simulink model "Demo_TrafficFlow_cs"  (Simulink 7.6)
settings: Solver=ode5  SolverMode=SingleTasking  StartTime=0.0  StopTime=60  FixedStep=1/20  RelTol=1e-3
PreLoadFcn:
  | check_NUTS_defined
  | try
  |     evalin('base', 'NUTS_defined;');
  | catch
  |     warningMsg = sprintf('PreScan Warning: \nUsing this model requires that MATLAB is configured for PreScan. The model may not display correctly.\n\n ==> Please open MATLAB with the dedicated buttons provided with PreScan');
  |     disp(warningMsg)
  |     warndlg(warningMsg,'WindowStyle','Modal');
  | end
  | 
  | model_closed = 0;
  | assignin('base',['model_closed_' bdroot],false);
InitFcn:
  | PreScanExperimentInitFcn(bdroot)
StopFcn:
  | PreScanExperimentStopFcn(bdroot)
undefined symbols (not defined in the file or in its folder): Advised_Speed_L1, Advised_Speed_L2, Advised_Speed_L3, DiscIntNeverNeededParam, spare_10, spare_5, spare_6, spare_7, spare_8, spare_9, State_L2, Throughput_L1, Throughput_L2, Throughput_L3, Velocity_L1, Velocity_L2, Velocity_L3
libraries: simulink x58, PreScanSimulinkLibrary x40, simulink_extras x32, PreScanUsefulBlocks x22, simulink_need_slupdate x3
(part 15 of 20)

== system Demo_TrafficFlow_cs/Citroen_C3_1/AIR_3_Demux/Signal2ArrayOfSignals2 ==
  note: Set mask parameter VECTORLENGTH
      |  to MAXMESSAGES
  note: Initial state of Previous vector block
      | set to zeros(VECTORLENGTH,1)
  LoopIndex : Inport
  Element : Inport  Port=2
  Assignment : Assignment  IndexOptions=Index vector (port)  Indices=1  OutputSizes=1
  Previous vector : Memory  X0=zeros(VECTORLENGTH,1)  InheritSampleTime=on
  ArrayofElements : Outport
  wires:
  Element/1 -> Assignment/2
  LoopIndex/1 -> Assignment/3
  Previous vector/1 -> Assignment/1
  Assignment/1 -> ArrayofElements/1, Previous vector/1

== system Demo_TrafficFlow_cs/Citroen_C3_1/AIR_3_Demux/Signal2ArrayOfSignals3 ==
  note: Set mask parameter VECTORLENGTH
      |  to MAXMESSAGES
  note: Initial state of Previous vector block
      | set to zeros(VECTORLENGTH,1)
  LoopIndex : Inport
  Element : Inport  Port=2
  Assignment : Assignment  IndexOptions=Index vector (port)  Indices=1  OutputSizes=1
  Previous vector : Memory  X0=zeros(VECTORLENGTH,1)  InheritSampleTime=on
  ArrayofElements : Outport
  wires:
  Element/1 -> Assignment/2
  LoopIndex/1 -> Assignment/3
  Previous vector/1 -> Assignment/1
  Assignment/1 -> ArrayofElements/1, Previous vector/1

== system Demo_TrafficFlow_cs/Citroen_C3_1/AIR_3_Demux/Signal2ArrayOfSignals4 ==
  note: Set mask parameter VECTORLENGTH
      |  to MAXMESSAGES
  note: Initial state of Previous vector block
      | set to zeros(VECTORLENGTH,1)
  LoopIndex : Inport
  Element : Inport  Port=2
  Assignment : Assignment  IndexOptions=Index vector (port)  Indices=1  OutputSizes=1
  Previous vector : Memory  X0=zeros(VECTORLENGTH,1)  InheritSampleTime=on
  ArrayofElements : Outport
  wires:
  Element/1 -> Assignment/2
  LoopIndex/1 -> Assignment/3
  Previous vector/1 -> Assignment/1
  Assignment/1 -> ArrayofElements/1, Previous vector/1

== system Demo_TrafficFlow_cs/Citroen_C3_1/AIR_3_Demux/Signal2ArrayOfSignals5 ==
  note: Set mask parameter VECTORLENGTH
      |  to MAXMESSAGES
  note: Initial state of Previous vector block
      | set to zeros(VECTORLENGTH,1)
  LoopIndex : Inport
  Element : Inport  Port=2
  Assignment : Assignment  IndexOptions=Index vector (port)  Indices=1  OutputSizes=1
  Previous vector : Memory  X0=zeros(VECTORLENGTH,1)  InheritSampleTime=on
  ArrayofElements : Outport
  wires:
  Element/1 -> Assignment/2
  LoopIndex/1 -> Assignment/3
  Previous vector/1 -> Assignment/1
  Assignment/1 -> ArrayofElements/1, Previous vector/1

== system Demo_TrafficFlow_cs/Citroen_C3_1/AIR_3_Demux/Signal2ArrayOfSignals6 ==
  note: Set mask parameter VECTORLENGTH
      |  to MAXMESSAGES
  note: Initial state of Previous vector block
      | set to zeros(VECTORLENGTH,1)
  LoopIndex : Inport
  Element : Inport  Port=2
  Assignment : Assignment  IndexOptions=Index vector (port)  Indices=1  OutputSizes=1
  Previous vector : Memory  X0=zeros(VECTORLENGTH,1)  InheritSampleTime=on
  ArrayofElements : Outport
  wires:
  Element/1 -> Assignment/2
  LoopIndex/1 -> Assignment/3
  Previous vector/1 -> Assignment/1
  Assignment/1 -> ArrayofElements/1, Previous vector/1

== system Demo_TrafficFlow_cs/Citroen_C3_1/RFOBU_3_Rx_Demux/Reorder ==
  In : Inport
  Selector1 : Selector  InputPortWidth=InputPortWidth  IndexOptions=Index vector (dialog)  Indices=Elements  OutputSizes=1
  Out : Outport
  wires:
  In/1 -> Selector1/1
  Selector1/1 -> Out/1

== system Demo_TrafficFlow_cs/Citroen_C3_1/SELF_Demux/compas converter ==
  rotz [rad] : Inport
  cart2compas : Subsystem
  rad2deg : Gain  Gain=180/pi
  heading [deg] : Outport
  wires:
  cart2compas/1(heading [deg]) -> heading [deg]/1
  rotz [rad]/1 -> rad2deg/1
  rad2deg/1 -> cart2compas/1(Angle [deg])

== system Demo_TrafficFlow_cs/Citroen_C3_1/Simple_Longitudinal_ACC/HOLD Advised Speed ==
  v : Inport
  Memory : Memory  X0=50
  Switch : Switch  Criteria=u2 > Threshold
  v_hold : Outport
  wires:
  v/1 -> Switch/1, Switch/2
  Memory/1 -> Switch/3
  Switch/1 -> v_hold/1, Memory/1

== system Demo_TrafficFlow_cs/Citroen_C3_1/Simple_Longitudinal_ACC/LONGITUDINAL ACC & Dynamics ==
  note: DYNAMICS
  note: CONTROL
  note: CASE: DETECTION
      | vehicle in front detected
      | * postion control
      | * velocity control
  note: CASE: NO DETECTION
      | no vehicle in front detected
      | * velocity control
  Detected Range [m] : Inport
  Detected Velocity Vehicle [m/s] : Inport  Port=2
  Own Velocity Intention [km/h] : Inport  Port=3
  Safety Distance [m] : Inport  Port=4
  Gain1 : Gain  Gain=0.35
  Gain2 : Gain  Gain=1/3.6
  Rate Limiter2 : RateLimiter  RisingSlewLimit=3  FallingSlewLimit=-3  SampleTimeMode=inherited
  Sum : Sum  Inputs=-+|
  Sum2 : Sum  Inputs=++|
  Switch : Switch  Threshold=0.0001
  only forward driving : Saturate  UpperLimit=inf  LowerLimit=0
  Velocity [m/s] : Outport
  wires:
  Rate Limiter2/1 -> only forward driving/1
  only forward driving/1 -> Velocity [m/s]/1
  Sum/1 -> Gain1/1  "Control Error"
  Switch/1 -> Rate Limiter2/1
  Detected Range [m]/1 -> Switch/2, Sum/2
  Own Velocity Intention [km/h]/1 -> Gain2/1
  Gain2/1 -> Switch/3
  Sum2/1 -> Switch/1
  Detected Velocity Vehicle [m/s]/1 -> Sum2/2
  Safety Distance [m]/1 -> Sum/1
  Gain1/1 -> Sum2/1

== system Demo_TrafficFlow_cs/Citroen_C3_1/Trajectories/END OF TRACK REACHED & GO TO BUFFER ==
  Elapsed_Track [m] : Inport
  Length_of_Track [m] : Inport  Port=2
  Product1 : Product  RndMeth=Zero
  Relational Operator : RelationalOperator  OutDataTypeStr=boolean
  offset for displacement to  buffer of traffic LANE 3 : Constant  Tag=TrajSubSelector  Value=[0 27 0 0 0 0 0 0 0]
  Buffer_offset [m] : Outport
  End_of_Track_Reached [-] : Outport  Port=2
  wires:
  offset for displacement to  buffer of traffic LANE 3/1 -> Product1/1
  Relational Operator/1 -> Product1/2, End_of_Track_Reached [-]/1
  Elapsed_Track [m]/1 -> Relational Operator/1
  Product1/1 -> Buffer_offset [m]/1
  Length_of_Track [m]/1 -> Relational Operator/2

== system Demo_TrafficFlow_cs/Citroen_C3_1/Trajectories/ElapsedTrack1 ==
  Offset : Inport
  s : Inport  Port=2
  Mux : Mux  Inputs=3
  Mux1 : Mux  Inputs=3
  MuxState : Subsystem
  OffsetCor : Subsystem
  pitchAngle : Lookup  InputValues=s  Table=pitch  LookUpMeth=Interpolation-Use End Values
  rollAngle : Lookup  InputValues=s  Table=roll  LookUpMeth=Interpolation-Use End Values
  xpos : Lookup  InputValues=s  Table=x  LookUpMeth=Interpolation-Use End Values
  yawAngle : Lookup  InputValues=s  Table=yaw  LookUpMeth=Interpolation-Use End Values
  ypos : Lookup  InputValues=s  Table=y  LookUpMeth=Interpolation-Use End Values
  zpos : Lookup  InputValues=s  Table=z  LookUpMeth=Interpolation-Use End Values
  state : Outport
  wires:
  zpos/1 -> OffsetCor/3(z pos)
  rollAngle/1 -> OffsetCor/4(R angle)
  pitchAngle/1 -> OffsetCor/5(P angle)
  yawAngle/1 -> OffsetCor/6(Y angle)
  OffsetCor/6(Y angle_cor) -> Mux1/3
  OffsetCor/5(P angle_cor) -> Mux1/2
  OffsetCor/4(R angle_cor) -> Mux1/1
  OffsetCor/3(z pos_cor) -> Mux/3
  MuxState/1(State) -> state/1
  Mux/1 -> MuxState/1(xyzPos_[m])
  s/1 -> pitchAngle/1, yawAngle/1, rollAngle/1, zpos/1, ypos/1, xpos/1
  Offset/1 -> OffsetCor/7(Offset)
  xpos/1 -> OffsetCor/1(x pos)
  ypos/1 -> OffsetCor/2(y pos)
  OffsetCor/1(x pos_cor) -> Mux/1
  OffsetCor/2(y pos_cor) -> Mux/2
  Mux1/1 -> MuxState/2(RPYAngle_[rad])

== system Demo_TrafficFlow_cs/Citroen_C3_1/Trajectories/LANE CHANGE LEFT (cummulative y position;  multiple shift to left are possible) ==
  reset cum value : Inport
  velocity [m/s] : Inport  Port=2
  on/off [-] : Inport  Port=3
  Cumulative Lane Change : Subsystem
  Single LaneChange_Left : Subsystem
  Trigger : Subsystem  TreatAsAtomicUnit=on
  cum LC : Outport
  wires:
  on/off [-]/1 -> Single LaneChange_Left/1(on/off [-]), Trigger/Trigger  "lane_change_signal"
  Single LaneChange_Left/1(end LC reached) -> Cumulative Lane Change/2(end LC reached)  "end_reached"
  Trigger/1(Out1) -> Single LaneChange_Left/Enable
  velocity [m/s]/1 -> Single LaneChange_Left/2(velocity [m/s])
  reset cum value/1 -> Cumulative Lane Change/1(reset cum value)  "reset_signal"
  Single LaneChange_Left/2(single LC value) -> Cumulative Lane Change/3(single LC value)  "single LC value"
  Cumulative Lane Change/1(cum LC value) -> cum LC/1

== system Demo_TrafficFlow_cs/Citroen_C3_1/Trajectories/PathFollowerSva_3 ==
  From Workspace : FromWorkspace  VariableName=trajectory  SampleTime=TimeStep  OutputAfterFinalValue=Holding final value
  From Workspace1 : FromWorkspace  VariableName=trajectory  SampleTime=TimeStep  OutputAfterFinalValue=Setting to zero
  Mux : Mux  Inputs=2
  Selector1 : Selector  InputPortWidth=24  IndexOptions=Index vector (dialog)  Indices=[7:18]  OutputSizes=1
  Selector9 : Selector  InputPortWidth=24  IndexOptions=Index vector (dialog)  Indices=[1:6]  OutputSizes=1
  trajectory : Outport
  wires:
  Selector1/1 -> Mux/2
  Selector9/1 -> Mux/1
  Mux/1 -> trajectory/1
  From Workspace1/1 -> Selector1/1
  From Workspace/1 -> Selector9/1

== system Demo_TrafficFlow_cs/Citroen_C3_1/Trajectories/RESTART TRAJECT ==
  End_of_Track_Reached [-] : Inport
  ID_start_again [-] : Inport  Port=2
  objectID [-] : Inport  Port=3
  Logical Operator : Logic  AllPortsSameDT=off  OutDataTypeStr=boolean
  Memory : Memory
  Relational Operator1 : RelationalOperator  Operator===  OutDataTypeStr=boolean
  reset [-] : Outport
  Start_Traject [-] : Outport  Port=2
  wires:
  objectID [-]/1 -> Relational Operator1/2
  Logical Operator/1 -> Memory/1
  End_of_Track_Reached [-]/1 -> Logical Operator/1
  Memory/1 -> Start_Traject [-]/1
  Relational Operator1/1 -> Logical Operator/2, reset [-]/1
  ID_start_again [-]/1 -> Relational Operator1/1

== system Demo_TrafficFlow_cs/Citroen_C3_1/Trajectories/Random  LC generator ==
  Random Number : RandomNumber  Mean=0.5  Variance=0.1  SampleTime=0
  Rounding Function : Rounding  Operator=round
  0/1 : Outport
  wires:
  Random Number/1 -> Rounding Function/1
  Rounding Function/1 -> 0/1/1

== system Demo_TrafficFlow_cs/Citroen_C3_1/Trajectories/Traj2State1 ==
  Traj Org : Inport
  Offset : Inport  Port=2
  Add2 : Sum
  ApplyRotation : PreScanSimulinkLibrary/ApplyRotation  SystemSampleTime=-1
  ApplyRotation1 : PreScanSimulinkLibrary/ApplyRotation  SystemSampleTime=-1
  ApplyRotation2 : PreScanSimulinkLibrary/ApplyRotation  SystemSampleTime=-1
  Constant : Constant  Value=zeros(12,1)
  Mux : Mux  Inputs=6
  Mux1 : Mux  Inputs=2
  Mux{2,4..9} (7 alike) : Mux  Inputs=3
  Mux3 : Mux  Inputs=2
  Selector : Selector  InputPortWidth=6  IndexOptions=Index vector (dialog)  Indices=[4 5 6]  OutputSizes=1
  Selector1 : Selector  InputPortWidth=21  IndexOptions=Index vector (dialog)  Indices=[5]  OutputSizes=1
  Selector10 : Selector  InputPortWidth=21  IndexOptions=Index vector (dialog)  Indices=[2]  OutputSizes=1
  Selector11 : Selector  InputPortWidth=21  IndexOptions=Index vector (dialog)  Indices=[3]  OutputSizes=1
  Selector12 : Selector  InputPortWidth=21  IndexOptions=Index vector (dialog)  Indices=[1]  OutputSizes=1
  Selector13 : Selector  InputPortWidth=21  IndexOptions=Index vector (dialog)  Indices=[8]  OutputSizes=1
  Selector14 : Selector  InputPortWidth=21  IndexOptions=Index vector (dialog)  Indices=[9]  OutputSizes=1
  Selector15 : Selector  InputPortWidth=21  IndexOptions=Index vector (dialog)  Indices=[7]  OutputSizes=1
  Selector16 : Selector  InputPortWidth=21  IndexOptions=Index vector (dialog)  Indices=[14]  OutputSizes=1
  Selector17 : Selector  InputPortWidth=21  IndexOptions=Index vector (dialog)  Indices=[15]  OutputSizes=1
  Selector18 : Selector  InputPortWidth=21  IndexOptions=Index vector (dialog)  Indices=[13]  OutputSizes=1
  Selector19 : Selector  InputPortWidth=21  IndexOptions=Index vector (dialog)  Indices=[20]  OutputSizes=1
  Selector2 : Selector  InputPortWidth=21  IndexOptions=Index vector (dialog)  Indices=[6]  OutputSizes=1
  Selector20 : Selector  InputPortWidth=21  IndexOptions=Index vector (dialog)  Indices=[21]  OutputSizes=1
  Selector21 : Selector  InputPortWidth=21  IndexOptions=Index vector (dialog)  Indices=[19]  OutputSizes=1
  Selector3 : Selector  InputPortWidth=21  IndexOptions=Index vector (dialog)  Indices=[12]  OutputSizes=1
  Selector4 : Selector  InputPortWidth=21  IndexOptions=Index vector (dialog)  Indices=[17]  OutputSizes=1
  Selector5 : Selector  InputPortWidth=21  IndexOptions=Index vector (dialog)  Indices=[18]  OutputSizes=1
  Selector6 : Selector  InputPortWidth=21  IndexOptions=Index vector (dialog)  Indices=[16]  OutputSizes=1
  Selector7 : Selector  InputPortWidth=21  IndexOptions=Index vector (dialog)  Indices=[11]  OutputSizes=1
  Selector8 : Selector  InputPortWidth=21  IndexOptions=Index vector (dialog)  Indices=[10]  OutputSizes=1
  Selector9 : Selector  InputPortWidth=21  IndexOptions=Index vector (dialog)  Indices=[4]  OutputSizes=1
  Traj2State2 : Subsystem
  state : Outport
  wires:
  Mux9/1 -> ApplyRotation2/2, ApplyRotation1/2, ApplyRotation/2
  Selector20/1 -> Mux9/3
  Selector19/1 -> Mux9/2
  Selector21/1 -> Mux9/1
  Selector5/1 -> Mux8/3
  Selector4/1 -> Mux8/2
  Selector6/1 -> Mux8/1
  Mux8/1 -> Mux/6
  Mux6/1 -> ApplyRotation2/1
  Mux7/1 -> Mux/4
  ApplyRotation2/1 -> Mux/5
  Selector3/1 -> Mux7/3
  Selector7/1 -> Mux7/2
  Selector8/1 -> Mux7/1
  Mux4/1 -> Mux/2
  Mux5/1 -> ApplyRotation1/1
  ApplyRotation1/1 -> Mux/3
  Mux2/1 -> ApplyRotation/1
  ApplyRotation/1 -> Mux/1
  Selector2/1 -> Mux4/3
  Selector1/1 -> Mux4/2
  Selector9/1 -> Mux4/1
  Selector17/1 -> Mux6/3
  Selector16/1 -> Mux6/2
  Selector18/1 -> Mux6/1
  Selector14/1 -> Mux5/3
  Selector13/1 -> Mux5/2
  Selector15/1 -> Mux5/1
  Selector11/1 -> Mux2/3
  Selector10/1 -> Mux2/2
  Selector12/1 -> Mux2/1
  Mux3/1 -> Selector8/1, Selector7/1, Selector3/1, Selector18/1, Selector16/1, Selector17/1, Selector6/1, Selector20/1, Selector19/1, Selector21/1, Selector5/1, Selector4/1, Selector14/1, Selector13/1, Selector15/1, Selector2/1, Selector1/1, Selector9/1, Selector12/1, Selector10/1, Selector11/1
  Mux1/1 -> Add2/2
  Constant/1 -> Mux1/2
  Add2/1 -> Traj2State2/1(traj)
  Selector/1 -> Mux3/2
  Traj Org/1 -> Mux3/1
  Offset/1 -> Mux1/1, Selector/1
  Mux/1 -> Add2/1
  Traj2State2/1(state) -> state/1

== system Demo_TrafficFlow_cs/Control_Unit/Antenna_Rx_7_Demux/Reorder ==
  In : Inport
  Selector1 : Selector  InputPortWidth=InputPortWidth  IndexOptions=Index vector (dialog)  Indices=Elements  OutputSizes=1
  Out : Outport
  wires:
  Selector1/1 -> Out/1
  In/1 -> Selector1/1

== system Demo_TrafficFlow_cs/Control_Unit/Antenna_Rx_7_Demux/compas converter ==
  rotz [rad] : Inport
  cart2compas : Subsystem
  rad2deg : Gain  Gain=180/pi
  heading [deg] : Outport
  wires:
  rad2deg/1 -> cart2compas/1(Angle [deg])
  rotz [rad]/1 -> rad2deg/1
  cart2compas/1(heading [deg]) -> heading [deg]/1

== system Demo_TrafficFlow_cs/Control_Unit/Antenna_Rx_8_Demux/Reorder ==
  In : Inport
  Selector1 : Selector  InputPortWidth=InputPortWidth  IndexOptions=Index vector (dialog)  Indices=Elements  OutputSizes=1
  Out : Outport
  wires:
  Selector1/1 -> Out/1
  In/1 -> Selector1/1

== system Demo_TrafficFlow_cs/Control_Unit/Antenna_Rx_8_Demux/compas converter ==
  rotz [rad] : Inport
  cart2compas : Subsystem
  rad2deg : Gain  Gain=180/pi
  heading [deg] : Outport
  wires:
  rad2deg/1 -> cart2compas/1(Angle [deg])
  rotz [rad]/1 -> rad2deg/1
  cart2compas/1(heading [deg]) -> heading [deg]/1

== system Demo_TrafficFlow_cs/Control_Unit/Antenna_Rx_9_Demux/Reorder ==
  In : Inport
  Selector1 : Selector  InputPortWidth=InputPortWidth  IndexOptions=Index vector (dialog)  Indices=Elements  OutputSizes=1
  Out : Outport
  wires:
  Selector1/1 -> Out/1
  In/1 -> Selector1/1

== system Demo_TrafficFlow_cs/Control_Unit/Antenna_Rx_9_Demux/compas converter ==
  rotz [rad] : Inport
  cart2compas : Subsystem
  rad2deg : Gain  Gain=180/pi
  heading [deg] : Outport
  wires:
  rad2deg/1 -> cart2compas/1(Angle [deg])
  rotz [rad]/1 -> rad2deg/1
  cart2compas/1(heading [deg]) -> heading [deg]/1

== system Demo_TrafficFlow_cs/Control_Unit/Control Lane2/Lane2 Closed ==
  IfAction : ActionPort  PropagateVarSize=During execution  ActionType=else
  State: Lane2 Closed : Constant  Value=0
  Out : Outport  OutDataTypeStr=double  InitialOutput=[0]
  wires:
  State: Lane2 Closed/1 -> Out/1

== system Demo_TrafficFlow_cs/Control_Unit/Control Lane2/Lane2 open for Lane1 ==
  note: To make sure that the lane is empty,
      | a delay is applied based on advised
      | velocity
  Close time : Inport
  IfAction : ActionPort  InitializeStates=reset  PropagateVarSize=During execution  ActionType=then
  "Close Lane2 for X seconds " : RelationalOperator  OutDataTypeStr=boolean
  Counter : Subsystem
  Product : Product  RndMeth=Zero
  State: Lane2 open for Lane1 : Constant
  Out : Outport  InitialOutput=[1]
  wires:
  Product/1 -> Out/1
  State: Lane2 open for Lane1/1 -> Product/1
  Counter/1(Out1) -> "Close Lane2 for X seconds "/1
  "Close Lane2 for X seconds "/1 -> Product/2
  Close time/1 -> "Close Lane2 for X seconds "/2

== system Demo_TrafficFlow_cs/Control_Unit/Control Lane2/Lane2 open for Lane3 ==
  note: To make sure that the lane is empty,
      | a delay is applied based on advised
      | velocity
  Close time : Inport
  IfAction : ActionPort  InitializeStates=reset  PropagateVarSize=During execution  ActionType=elseif
  "Close Lane2 for X seconds " : RelationalOperator  OutDataTypeStr=boolean
  Counter : Subsystem
  Product : Product  RndMeth=Zero
  Scope : Scope  NumInputPorts=3
      settings: YMin=-5~-5~-5  YMax=5~5~5
  State: Lane2 open for Lane3 : Constant  Value=3
  Out : Outport  InitialOutput=[3]
  wires:
  "Close Lane2 for X seconds "/1 -> Product/2, Scope/2
  Product/1 -> Out/1
  Counter/1(Out1) -> "Close Lane2 for X seconds "/1, Scope/3
  State: Lane2 open for Lane3/1 -> Product/1
  Close time/1 -> "Close Lane2 for X seconds "/2, Scope/1

== system Demo_TrafficFlow_cs/HighwayOverhang_1/Antenna_Rx_2_Demux/Reorder ==
  In : Inport
  Selector1 : Selector  InputPortWidth=InputPortWidth  IndexOptions=Index vector (dialog)  Indices=Elements  OutputSizes=1
  Out : Outport
  wires:
  Selector1/1 -> Out/1
  In/1 -> Selector1/1

== system Demo_TrafficFlow_cs/HighwayOverhang_1/Antenna_Rx_2_Demux/compas converter ==
  rotz [rad] : Inport
  cart2compas : Subsystem
  rad2deg : Gain  Gain=180/pi
  heading [deg] : Outport
  wires:
  rad2deg/1 -> cart2compas/1(Angle [deg])
  rotz [rad]/1 -> rad2deg/1
  cart2compas/1(heading [deg]) -> heading [deg]/1

== system Demo_TrafficFlow_cs/HighwayOverhang_1/RFBeacon_1_Rx_Demux/Reorder ==
  In : Inport
  Selector1 : Selector  InputPortWidth=InputPortWidth  IndexOptions=Index vector (dialog)  Indices=Elements  OutputSizes=1
  Out : Outport
  wires:
  Selector1/1 -> Out/1
  In/1 -> Selector1/1

== system Demo_TrafficFlow_cs/HighwayOverhang_1/RFBeacon_1_Rx_Demux/compas converter ==
  rotz [rad] : Inport
  cart2compas : Subsystem
  rad2deg : Gain  Gain=180/pi
  heading [deg] : Outport
  wires:
  rad2deg/1 -> cart2compas/1(Angle [deg])
  rotz [rad]/1 -> rad2deg/1
  cart2compas/1(heading [deg]) -> heading [deg]/1

== system Demo_TrafficFlow_cs/HighwayOverhang_1/Throughput detection  Sensor/TrafficDensityCounter [cars/hour] ==
  Detection : Inport
  Velocity [m/s] : Inport  Port=2
  Gain1 : Gain  Gain=60/MT
  Gain2 : Gain  Gain=1/MT
  Gain3 : Gain  Gain=60*60/MT
  Increment Counter : Sum  Inputs=+-
  Memory : Memory
  Product1 : Product  RndMeth=Zero
  Product2 : Product  RndMeth=Zero
  Rate Transition : RateTransition
  Rate Transition1 : RateTransition  OutPortSampleTime=1/20
  Ref_Range1 : Constant  Value=0
  Relational Operator : RelationalOperator  Operator=>  OutDataTypeStr=boolean
  Relational Operator1 : RelationalOperator  Operator=~=  OutDataTypeStr=boolean
  Step1 : Step  Time=MT*0  SampleTime=0
  Subsystem : Subsystem
  Subsystem1 : Subsystem
  Transport Delay1 : TransportDelay  DelayTime=MT
  Weighted Moving Average : simulink_need_slupdate/Weighted Moving Average  mgainval=ones(1,MT*20)/(MT*20)  vinit=0.0  samptime=1/20  OutDataType=sfix(16)  OutScaling=2^-10  DoSatur=off  GainDataType=sfix( 16 )  MatRadixGroup=Best Precision: Matrix-wise  GainScaling=2^-10
  Out_[1/MT]_unweighted : Outport
  Out_[1/MT] : Outport  Port=2
  Out_[1/s] : Outport  Port=3
  Out_[1/min] : Outport  Port=4
  Out_[1/hour] : Outport  Port=5
  Velocity [km/h] : Outport  Port=6
  wires:
  Ref_Range1/1 -> Relational Operator/2
  Detection/1 -> Relational Operator/1, Relational Operator1/2, Memory/1
  Subsystem/1(Out1) -> Transport Delay1/1, Increment Counter/1
  Transport Delay1/1 -> Increment Counter/2
  Increment Counter/1 -> Product1/2
  Step1/1 -> Product1/1
  Product1/1 -> Rate Transition1/1, Out_[1/MT]_unweighted/1
  Relational Operator1/1 -> Product2/1
  Relational Operator/1 -> Product2/2
  Memory/1 -> Relational Operator1/1
  Product2/1 -> Subsystem/Enable, Subsystem1/Enable
  Weighted Moving Average/1 -> Rate Transition/1
  Rate Transition/1 -> Out_[1/MT]/1, Gain2/1, Gain1/1, Gain3/1
  Rate Transition1/1 -> Weighted Moving Average/1
  Gain2/1 -> Out_[1/s]/1
  Gain1/1 -> Out_[1/min]/1
  Gain3/1 -> Out_[1/hour]/1
  Velocity [m/s]/1 -> Subsystem1/1(Velocity [m/s])
  Subsystem1/1(Velocity [km/h]) -> Velocity [km/h]/1

== system Demo_TrafficFlow_cs/HighwayOverhang_2/Antenna_Rx_3_Demux/Reorder ==
  In : Inport
  Selector1 : Selector  InputPortWidth=InputPortWidth  IndexOptions=Index vector (dialog)  Indices=Elements  OutputSizes=1
  Out : Outport
  wires:
  Selector1/1 -> Out/1
  In/1 -> Selector1/1

== system Demo_TrafficFlow_cs/HighwayOverhang_2/Antenna_Rx_3_Demux/compas converter ==
  rotz [rad] : Inport
  cart2compas : Subsystem
  rad2deg : Gain  Gain=180/pi
  heading [deg] : Outport
  wires:
  rad2deg/1 -> cart2compas/1(Angle [deg])
  rotz [rad]/1 -> rad2deg/1
  cart2compas/1(heading [deg]) -> heading [deg]/1

== system Demo_TrafficFlow_cs/HighwayOverhang_2/Control Lane2/Lane2 Closed ==
  IfAction : ActionPort  PropagateVarSize=During execution  ActionType=else
  Closed for Lane 3 : Constant  Value=0
  Closed for Lane1 : Constant  Value=0
  Mux : Mux  Inputs=2
  Out : Outport  InitialOutput=[0 0]
  wires:
  Mux/1 -> Out/1
  Closed for Lane1/1 -> Mux/1
  Closed for Lane 3/1 -> Mux/2

== system Demo_TrafficFlow_cs/HighwayOverhang_2/Control Lane2/Lane2 open for Lane1 ==
  IfAction : ActionPort  InitializeStates=reset  PropagateVarSize=During execution  ActionType=then
  Closed for Lane 3 : Constant  Value=0
  Mux : Mux  Inputs=2
  Open for Lane1 : Constant
  Out : Outport  InitialOutput=[1 0 ]
  wires:
  Mux/1 -> Out/1
  Open for Lane1/1 -> Mux/1
  Closed for Lane 3/1 -> Mux/2

== system Demo_TrafficFlow_cs/HighwayOverhang_2/Control Lane2/Lane2 open for Lane3 ==
  IfAction : ActionPort  InitializeStates=reset  PropagateVarSize=During execution  ActionType=elseif
  Closed for Lane1 : Constant  Value=0
  Mux : Mux  Inputs=2
  Open for Lane 3 : Constant
  Out : Outport  InitialOutput=[0 1]
  wires:
  Mux/1 -> Out/1
  Closed for Lane1/1 -> Mux/1
  Open for Lane 3/1 -> Mux/2

== system Demo_TrafficFlow_cs/HighwayOverhang_2/RFBeacon_2_Rx_Demux/Reorder ==
  In : Inport
  Selector1 : Selector  InputPortWidth=InputPortWidth  IndexOptions=Index vector (dialog)  Indices=Elements  OutputSizes=1
  Out : Outport
  wires:
  Selector1/1 -> Out/1
  In/1 -> Selector1/1

== system Demo_TrafficFlow_cs/HighwayOverhang_2/RFBeacon_2_Rx_Demux/compas converter ==
  rotz [rad] : Inport
  cart2compas : Subsystem
  rad2deg : Gain  Gain=180/pi
  heading [deg] : Outport
  wires:
  rad2deg/1 -> cart2compas/1(Angle [deg])
  rotz [rad]/1 -> rad2deg/1
  cart2compas/1(heading [deg]) -> heading [deg]/1

== system Demo_TrafficFlow_cs/HighwayOverhang_2/Throughput detection  Sensor/TrafficDensityCounter [cars/hour] ==
  Detection : Inport
  Velocity [m/s] : Inport  Port=2
  Gain1 : Gain  Gain=60/MT
  Gain2 : Gain  Gain=1/MT
  Gain3 : Gain  Gain=60*60/MT
  Increment Counter : Sum  Inputs=+-
  Memory : Memory
  Product1 : Product  RndMeth=Zero
  Product2 : Product  RndMeth=Zero
  Rate Transition : RateTransition
  Rate Transition1 : RateTransition  OutPortSampleTime=1/20
  Ref_Range1 : Constant  Value=0
  Relational Operator : RelationalOperator  Operator=>  OutDataTypeStr=boolean
  Relational Operator1 : RelationalOperator  Operator=~=  OutDataTypeStr=boolean
  Step1 : Step  Time=MT*0  SampleTime=0
  Subsystem : Subsystem
  Subsystem1 : Subsystem
  Transport Delay1 : TransportDelay  DelayTime=MT
  Weighted Moving Average : simulink_need_slupdate/Weighted Moving Average  mgainval=ones(1,MT*20)/(MT*20)  vinit=0.0  samptime=1/20  OutDataType=sfix(16)  OutScaling=2^-10  DoSatur=off  GainDataType=sfix( 16 )  MatRadixGroup=Best Precision: Matrix-wise  GainScaling=2^-10
  Out_[1/MT]_unweighted : Outport
  Out_[1/MT] : Outport  Port=2
  Out_[1/s] : Outport  Port=3
  Out_[1/min] : Outport  Port=4
  Out_[1/hour] : Outport  Port=5
  Velocity [km/h] : Outport  Port=6
  wires:
  Ref_Range1/1 -> Relational Operator/2
  Detection/1 -> Relational Operator/1, Relational Operator1/2, Memory/1
  Subsystem/1(Out1) -> Transport Delay1/1, Increment Counter/1
  Transport Delay1/1 -> Increment Counter/2
  Increment Counter/1 -> Product1/2
  Step1/1 -> Product1/1
  Product1/1 -> Rate Transition1/1, Out_[1/MT]_unweighted/1
  Relational Operator1/1 -> Product2/1
  Relational Operator/1 -> Product2/2
  Memory/1 -> Relational Operator1/1
  Product2/1 -> Subsystem/Enable, Subsystem1/Enable
  Weighted Moving Average/1 -> Rate Transition/1
  Rate Transition/1 -> Out_[1/MT]/1, Gain2/1, Gain1/1, Gain3/1
  Rate Transition1/1 -> Weighted Moving Average/1
  Gain2/1 -> Out_[1/s]/1
  Gain1/1 -> Out_[1/min]/1
  Gain3/1 -> Out_[1/hour]/1
  Velocity [m/s]/1 -> Subsystem1/1(Velocity [m/s])
  Subsystem1/1(Velocity [km/h]) -> Velocity [km/h]/1

== system Demo_TrafficFlow_cs/HighwayOverhang_3/Antenna_Rx_4_Demux/Reorder ==
  In : Inport
  Selector1 : Selector  InputPortWidth=InputPortWidth  IndexOptions=Index vector (dialog)  Indices=Elements  OutputSizes=1
  Out : Outport
  wires:
  Selector1/1 -> Out/1
  In/1 -> Selector1/1

== system Demo_TrafficFlow_cs/HighwayOverhang_3/Antenna_Rx_4_Demux/compas converter ==
  rotz [rad] : Inport
  cart2compas : Subsystem
  rad2deg : Gain  Gain=180/pi
  heading [deg] : Outport
  wires:
  rad2deg/1 -> cart2compas/1(Angle [deg])
  rotz [rad]/1 -> rad2deg/1
  cart2compas/1(heading [deg]) -> heading [deg]/1

== system Demo_TrafficFlow_cs/HighwayOverhang_3/RFBeacon_3_Rx_Demux/Reorder ==
  In : Inport
  Selector1 : Selector  InputPortWidth=InputPortWidth  IndexOptions=Index vector (dialog)  Indices=Elements  OutputSizes=1
  Out : Outport
  wires:
  Selector1/1 -> Out/1
  In/1 -> Selector1/1

== system Demo_TrafficFlow_cs/HighwayOverhang_3/RFBeacon_3_Rx_Demux/compas converter ==
  rotz [rad] : Inport
  cart2compas : Subsystem
  rad2deg : Gain  Gain=180/pi
  heading [deg] : Outport
  wires:
  rad2deg/1 -> cart2compas/1(Angle [deg])
  rotz [rad]/1 -> rad2deg/1
  cart2compas/1(heading [deg]) -> heading [deg]/1

== system Demo_TrafficFlow_cs/HighwayOverhang_3/Throughput detection  Sensor/TrafficDensityCounter [cars/hour] ==
  Detection : Inport
  Velocity [m/s] : Inport  Port=2
  Gain1 : Gain  Gain=60/MT
  Gain2 : Gain  Gain=1/MT
  Gain3 : Gain  Gain=60*60/MT
  Increment Counter : Sum  Inputs=+-
  Memory : Memory
  Product1 : Product  RndMeth=Zero
  Product2 : Product  RndMeth=Zero
  Rate Transition : RateTransition
  Rate Transition1 : RateTransition  OutPortSampleTime=1/20
  Ref_Range1 : Constant  Value=0
  Relational Operator : RelationalOperator  Operator=>  OutDataTypeStr=boolean
  Relational Operator1 : RelationalOperator  Operator=~=  OutDataTypeStr=boolean
  Step1 : Step  Time=MT*0  SampleTime=0
  Subsystem : Subsystem
  Subsystem1 : Subsystem
  Transport Delay1 : TransportDelay  DelayTime=MT
  Weighted Moving Average : simulink_need_slupdate/Weighted Moving Average  mgainval=ones(1,MT*20)/(MT*20)  vinit=0.0  samptime=1/20  OutDataType=sfix(16)  OutScaling=2^-10  DoSatur=off  GainDataType=sfix( 16 )  MatRadixGroup=Best Precision: Matrix-wise  GainScaling=2^-10
  Out_[1/MT]_unweighted : Outport
  Out_[1/MT] : Outport  Port=2
  Out_[1/s] : Outport  Port=3
  Out_[1/min] : Outport  Port=4
  Out_[1/hour] : Outport  Port=5
  Velocity [km/h] : Outport  Port=6
  wires:
  Ref_Range1/1 -> Relational Operator/2
  Detection/1 -> Relational Operator/1, Relational Operator1/2, Memory/1
  Subsystem/1(Out1) -> Transport Delay1/1, Increment Counter/1
  Transport Delay1/1 -> Increment Counter/2
  Increment Counter/1 -> Product1/2
  Step1/1 -> Product1/1
  Product1/1 -> Rate Transition1/1, Out_[1/MT]_unweighted/1
  Relational Operator1/1 -> Product2/1
  Relational Operator/1 -> Product2/2
  Memory/1 -> Relational Operator1/1
  Product2/1 -> Subsystem/Enable, Subsystem1/Enable
  Weighted Moving Average/1 -> Rate Transition/1
  Rate Transition/1 -> Out_[1/MT]/1, Gain2/1, Gain1/1, Gain3/1
  Rate Transition1/1 -> Weighted Moving Average/1
  Gain2/1 -> Out_[1/s]/1
  Gain1/1 -> Out_[1/min]/1
  Gain3/1 -> Out_[1/hour]/1
  Velocity [m/s]/1 -> Subsystem1/1(Velocity [m/s])
  Subsystem1/1(Velocity [km/h]) -> Velocity [km/h]/1

== system Demo_TrafficFlow_cs/Mazda_RX8_1/AIR_4_Demux/Activate_Switch ==
  BeamID : Inport
  Heading : Inport  Port=2
  Constant : Constant  Value=0
  Product1 : Product  RndMeth=Zero
  Relational Operator : RelationalOperator  Operator=~=  OutDataTypeStr=boolean
  On/Off : Outport
  wires:
  Constant/1 -> Relational Operator/1
  Relational Operator/1 -> Product1/1
  BeamID/1 -> Relational Operator/2
  Product1/1 -> On/Off/1
  Heading/1 -> Product1/2

== system Demo_TrafficFlow_cs/Mazda_RX8_1/AIR_4_Demux/Signal2ArrayOfSignals1 ==
  note: Set mask parameter VECTORLENGTH
      |  to MAXMESSAGES
  note: Initial state of Previous vector block
      | set to zeros(VECTORLENGTH,1)
  LoopIndex : Inport
  Element : Inport  Port=2
  Assignment : Assignment  IndexOptions=Index vector (port)  Indices=1  OutputSizes=1
  Previous vector : Memory  X0=zeros(VECTORLENGTH,1)  InheritSampleTime=on
  ArrayofElements : Outport
  wires:
  Element/1 -> Assignment/2
  LoopIndex/1 -> Assignment/3
  Previous vector/1 -> Assignment/1
  Assignment/1 -> ArrayofElements/1, Previous vector/1

== system Demo_TrafficFlow_cs/Mazda_RX8_1/AIR_4_Demux/Signal2ArrayOfSignals2 ==
  note: Set mask parameter VECTORLENGTH
      |  to MAXMESSAGES
  note: Initial state of Previous vector block
      | set to zeros(VECTORLENGTH,1)
  LoopIndex : Inport
  Element : Inport  Port=2
  Assignment : Assignment  IndexOptions=Index vector (port)  Indices=1  OutputSizes=1
  Previous vector : Memory  X0=zeros(VECTORLENGTH,1)  InheritSampleTime=on
  ArrayofElements : Outport
  wires:
  Element/1 -> Assignment/2
  LoopIndex/1 -> Assignment/3
  Previous vector/1 -> Assignment/1
  Assignment/1 -> ArrayofElements/1, Previous vector/1

== system Demo_TrafficFlow_cs/Mazda_RX8_1/AIR_4_Demux/Signal2ArrayOfSignals3 ==
  note: Set mask parameter VECTORLENGTH
      |  to MAXMESSAGES
  note: Initial state of Previous vector block
      | set to zeros(VECTORLENGTH,1)
  LoopIndex : Inport
  Element : Inport  Port=2
  Assignment : Assignment  IndexOptions=Index vector (port)  Indices=1  OutputSizes=1
  Previous vector : Memory  X0=zeros(VECTORLENGTH,1)  InheritSampleTime=on
  ArrayofElements : Outport
  wires:
  Element/1 -> Assignment/2
  LoopIndex/1 -> Assignment/3
  Previous vector/1 -> Assignment/1
  Assignment/1 -> ArrayofElements/1, Previous vector/1

== system Demo_TrafficFlow_cs/Mazda_RX8_1/AIR_4_Demux/Signal2ArrayOfSignals4 ==
  note: Set mask parameter VECTORLENGTH
      |  to MAXMESSAGES
  note: Initial state of Previous vector block
      | set to zeros(VECTORLENGTH,1)
  LoopIndex : Inport
  Element : Inport  Port=2
  Assignment : Assignment  IndexOptions=Index vector (port)  Indices=1  OutputSizes=1
  Previous vector : Memory  X0=zeros(VECTORLENGTH,1)  InheritSampleTime=on
  ArrayofElements : Outport
  wires:
  Element/1 -> Assignment/2
  LoopIndex/1 -> Assignment/3
  Previous vector/1 -> Assignment/1
  Assignment/1 -> ArrayofElements/1, Previous vector/1

== system Demo_TrafficFlow_cs/Mazda_RX8_1/AIR_4_Demux/Signal2ArrayOfSignals5 ==
  note: Set mask parameter VECTORLENGTH
      |  to MAXMESSAGES
  note: Initial state of Previous vector block
      | set to zeros(VECTORLENGTH,1)
  LoopIndex : Inport
  Element : Inport  Port=2
  Assignment : Assignment  IndexOptions=Index vector (port)  Indices=1  OutputSizes=1
  Previous vector : Memory  X0=zeros(VECTORLENGTH,1)  InheritSampleTime=on
  ArrayofElements : Outport
  wires:
  Element/1 -> Assignment/2
  LoopIndex/1 -> Assignment/3
  Previous vector/1 -> Assignment/1
  Assignment/1 -> ArrayofElements/1, Previous vector/1

== system Demo_TrafficFlow_cs/Mazda_RX8_1/AIR_4_Demux/Signal2ArrayOfSignals6 ==
  note: Set mask parameter VECTORLENGTH
      |  to MAXMESSAGES
  note: Initial state of Previous vector block
      | set to zeros(VECTORLENGTH,1)
  LoopIndex : Inport
  Element : Inport  Port=2
  Assignment : Assignment  IndexOptions=Index vector (port)  Indices=1  OutputSizes=1
  Previous vector : Memory  X0=zeros(VECTORLENGTH,1)  InheritSampleTime=on
  ArrayofElements : Outport
  wires:
  Element/1 -> Assignment/2
  LoopIndex/1 -> Assignment/3
  Previous vector/1 -> Assignment/1
  Assignment/1 -> ArrayofElements/1, Previous vector/1

== system Demo_TrafficFlow_cs/Mazda_RX8_1/RFOBU_4_Rx_Demux/Reorder ==
  In : Inport
  Selector1 : Selector  InputPortWidth=InputPortWidth  IndexOptions=Index vector (dialog)  Indices=Elements  OutputSizes=1
  Out : Outport
  wires:
  In/1 -> Selector1/1
  Selector1/1 -> Out/1

== system Demo_TrafficFlow_cs/Mazda_RX8_1/SELF_Demux/compas converter ==
  rotz [rad] : Inport
  cart2compas : Subsystem
  rad2deg : Gain  Gain=180/pi
  heading [deg] : Outport
  wires:
  cart2compas/1(heading [deg]) -> heading [deg]/1
  rotz [rad]/1 -> rad2deg/1
  rad2deg/1 -> cart2compas/1(Angle [deg])

== system Demo_TrafficFlow_cs/Mazda_RX8_1/Simple_Longitudinal_ACC/HOLD Advised Speed ==
  v : Inport
  Memory : Memory  X0=50
  Switch : Switch  Criteria=u2 > Threshold
  v_hold : Outport
  wires:
  v/1 -> Switch/1, Switch/2
  Memory/1 -> Switch/3
  Switch/1 -> v_hold/1, Memory/1

== system Demo_TrafficFlow_cs/Mazda_RX8_1/Simple_Longitudinal_ACC/LONGITUDINAL ACC & Dynamics ==
  note: DYNAMICS
  note: CONTROL
  note: CASE: DETECTION
      | vehicle in front detected
      | * postion control
      | * velocity control
  note: CASE: NO DETECTION
      | no vehicle in front detected
      | * velocity control
  Detected Range [m] : Inport
  Detected Velocity Vehicle [m/s] : Inport  Port=2
  Own Velocity Intention [km/h] : Inport  Port=3
  Safety Distance [m] : Inport  Port=4
  Gain1 : Gain  Gain=0.35
  Gain2 : Gain  Gain=1/3.6
  Rate Limiter2 : RateLimiter  RisingSlewLimit=3  FallingSlewLimit=-3  SampleTimeMode=inherited
  Sum : Sum  Inputs=-+|
  Sum2 : Sum  Inputs=++|
  Switch : Switch  Threshold=0.0001
  only forward driving : Saturate  UpperLimit=inf  LowerLimit=0
  Velocity [m/s] : Outport
  wires:
  Rate Limiter2/1 -> only forward driving/1
  only forward driving/1 -> Velocity [m/s]/1
  Sum/1 -> Gain1/1  "Control Error"
  Switch/1 -> Rate Limiter2/1
  Detected Range [m]/1 -> Switch/2, Sum/2
  Own Velocity Intention [km/h]/1 -> Gain2/1
  Gain2/1 -> Switch/3
  Sum2/1 -> Switch/1
  Detected Velocity Vehicle [m/s]/1 -> Sum2/2
  Safety Distance [m]/1 -> Sum/1
  Gain1/1 -> Sum2/1

== system Demo_TrafficFlow_cs/Mazda_RX8_1/Trajectories/END OF TRACK REACHED & GO TO BUFFER ==
  Elapsed_Track [m] : Inport
  Length_of_Track [m] : Inport  Port=2
  Product1 : Product  RndMeth=Zero
  Relational Operator : RelationalOperator  OutDataTypeStr=boolean
  offset for displacement to  buffer of traffic LANE 3 : Constant  Tag=TrajSubSelector  Value=[0 27 0 0 0 0 0 0 0]
  Buffer_offset [m] : Outport
  End_of_Track_Reached [-] : Outport  Port=2
  wires:
  offset for displacement to  buffer of traffic LANE 3/1 -> Product1/1
  Relational Operator/1 -> Product1/2, End_of_Track_Reached [-]/1
  Elapsed_Track [m]/1 -> Relational Operator/1
  Product1/1 -> Buffer_offset [m]/1
  Length_of_Track [m]/1 -> Relational Operator/2

== system Demo_TrafficFlow_cs/Mazda_RX8_1/Trajectories/ElapsedTrack1 ==
  Offset : Inport
  s : Inport  Port=2
  Mux : Mux  Inputs=3
  Mux1 : Mux  Inputs=3
  MuxState : Subsystem
  OffsetCor : Subsystem
  pitchAngle : Lookup  InputValues=s  Table=pitch  LookUpMeth=Interpolation-Use End Values
  rollAngle : Lookup  InputValues=s  Table=roll  LookUpMeth=Interpolation-Use End Values
  xpos : Lookup  InputValues=s  Table=x  LookUpMeth=Interpolation-Use End Values
  yawAngle : Lookup  InputValues=s  Table=yaw  LookUpMeth=Interpolation-Use End Values
  ypos : Lookup  InputValues=s  Table=y  LookUpMeth=Interpolation-Use End Values
  zpos : Lookup  InputValues=s  Table=z  LookUpMeth=Interpolation-Use End Values
  state : Outport
  wires:
  zpos/1 -> OffsetCor/3(z pos)
  rollAngle/1 -> OffsetCor/4(R angle)
  pitchAngle/1 -> OffsetCor/5(P angle)
  yawAngle/1 -> OffsetCor/6(Y angle)
  OffsetCor/6(Y angle_cor) -> Mux1/3
  OffsetCor/5(P angle_cor) -> Mux1/2
  OffsetCor/4(R angle_cor) -> Mux1/1
  OffsetCor/3(z pos_cor) -> Mux/3
  MuxState/1(State) -> state/1
  Mux/1 -> MuxState/1(xyzPos_[m])
  s/1 -> pitchAngle/1, yawAngle/1, rollAngle/1, zpos/1, ypos/1, xpos/1
  Offset/1 -> OffsetCor/7(Offset)
  xpos/1 -> OffsetCor/1(x pos)
  ypos/1 -> OffsetCor/2(y pos)
  OffsetCor/1(x pos_cor) -> Mux/1
  OffsetCor/2(y pos_cor) -> Mux/2
  Mux1/1 -> MuxState/2(RPYAngle_[rad])

== system Demo_TrafficFlow_cs/Mazda_RX8_1/Trajectories/LANE CHANGE LEFT (cummulative y position;  multiple shift to left are possible) ==
  reset cum value : Inport
  velocity [m/s] : Inport  Port=2
  on/off [-] : Inport  Port=3
  Cumulative Lane Change : Subsystem
  Single LaneChange_Left : Subsystem
  Trigger : Subsystem  TreatAsAtomicUnit=on
  cum LC : Outport
  wires:
  on/off [-]/1 -> Single LaneChange_Left/1(on/off [-]), Trigger/Trigger  "lane_change_signal"
  Single LaneChange_Left/1(end LC reached) -> Cumulative Lane Change/2(end LC reached)  "end_reached"
  Trigger/1(Out1) -> Single LaneChange_Left/Enable
  velocity [m/s]/1 -> Single LaneChange_Left/2(velocity [m/s])
  reset cum value/1 -> Cumulative Lane Change/1(reset cum value)  "reset_signal"
  Single LaneChange_Left/2(single LC value) -> Cumulative Lane Change/3(single LC value)  "single LC value"
  Cumulative Lane Change/1(cum LC value) -> cum LC/1

== system Demo_TrafficFlow_cs/Mazda_RX8_1/Trajectories/PathFollowerSva_4 ==
  From Workspace : FromWorkspace  VariableName=trajectory  SampleTime=TimeStep  OutputAfterFinalValue=Holding final value
  From Workspace1 : FromWorkspace  VariableName=trajectory  SampleTime=TimeStep  OutputAfterFinalValue=Setting to zero
  Mux : Mux  Inputs=2
  Selector1 : Selector  InputPortWidth=24  IndexOptions=Index vector (dialog)  Indices=[7:18]  OutputSizes=1
  Selector9 : Selector  InputPortWidth=24  IndexOptions=Index vector (dialog)  Indices=[1:6]  OutputSizes=1
  trajectory : Outport
  wires:
  Selector1/1 -> Mux/2
  Selector9/1 -> Mux/1
  Mux/1 -> trajectory/1
  From Workspace1/1 -> Selector1/1
  From Workspace/1 -> Selector9/1

== system Demo_TrafficFlow_cs/Mazda_RX8_1/Trajectories/RESTART TRAJECT ==
  End_of_Track_Reached [-] : Inport
  ID_start_again [-] : Inport  Port=2
  objectID [-] : Inport  Port=3
  Logical Operator : Logic  AllPortsSameDT=off  OutDataTypeStr=boolean
  Memory : Memory
  Relational Operator1 : RelationalOperator  Operator===  OutDataTypeStr=boolean
  reset [-] : Outport
  Start_Traject [-] : Outport  Port=2
  wires:
  objectID [-]/1 -> Relational Operator1/2
  Logical Operator/1 -> Memory/1
  End_of_Track_Reached [-]/1 -> Logical Operator/1
  Memory/1 -> Start_Traject [-]/1
  Relational Operator1/1 -> Logical Operator/2, reset [-]/1
  ID_start_again [-]/1 -> Relational Operator1/1

== system Demo_TrafficFlow_cs/Mazda_RX8_1/Trajectories/Random  LC generator ==
  Random Number : RandomNumber  Mean=0.5  Variance=0.1  SampleTime=0
  Rounding Function : Rounding  Operator=round
  0/1 : Outport
  wires:
  Random Number/1 -> Rounding Function/1
  Rounding Function/1 -> 0/1/1

== system Demo_TrafficFlow_cs/Mazda_RX8_1/Trajectories/Traj2State1 ==
  Traj Org : Inport
  Offset : Inport  Port=2
  Add2 : Sum
  ApplyRotation : PreScanSimulinkLibrary/ApplyRotation  SystemSampleTime=-1
  ApplyRotation1 : PreScanSimulinkLibrary/ApplyRotation  SystemSampleTime=-1
  ApplyRotation2 : PreScanSimulinkLibrary/ApplyRotation  SystemSampleTime=-1
  Constant : Constant  Value=zeros(12,1)
  Mux : Mux  Inputs=6
  Mux1 : Mux  Inputs=2
  Mux{2,4..9} (7 alike) : Mux  Inputs=3
  Mux3 : Mux  Inputs=2
  Selector : Selector  InputPortWidth=6  IndexOptions=Index vector (dialog)  Indices=[4 5 6]  OutputSizes=1
  Selector1 : Selector  InputPortWidth=21  IndexOptions=Index vector (dialog)  Indices=[5]  OutputSizes=1
  Selector10 : Selector  InputPortWidth=21  IndexOptions=Index vector (dialog)  Indices=[2]  OutputSizes=1
  Selector11 : Selector  InputPortWidth=21  IndexOptions=Index vector (dialog)  Indices=[3]  OutputSizes=1
  Selector12 : Selector  InputPortWidth=21  IndexOptions=Index vector (dialog)  Indices=[1]  OutputSizes=1
  Selector13 : Selector  InputPortWidth=21  IndexOptions=Index vector (dialog)  Indices=[8]  OutputSizes=1
  Selector14 : Selector  InputPortWidth=21  IndexOptions=Index vector (dialog)  Indices=[9]  OutputSizes=1
  Selector15 : Selector  InputPortWidth=21  IndexOptions=Index vector (dialog)  Indices=[7]  OutputSizes=1
  Selector16 : Selector  InputPortWidth=21  IndexOptions=Index vector (dialog)  Indices=[14]  OutputSizes=1
  Selector17 : Selector  InputPortWidth=21  IndexOptions=Index vector (dialog)  Indices=[15]  OutputSizes=1
  Selector18 : Selector  InputPortWidth=21  IndexOptions=Index vector (dialog)  Indices=[13]  OutputSizes=1
  Selector19 : Selector  InputPortWidth=21  IndexOptions=Index vector (dialog)  Indices=[20]  OutputSizes=1
  Selector2 : Selector  InputPortWidth=21  IndexOptions=Index vector (dialog)  Indices=[6]  OutputSizes=1
  Selector20 : Selector  InputPortWidth=21  IndexOptions=Index vector (dialog)  Indices=[21]  OutputSizes=1
  Selector21 : Selector  InputPortWidth=21  IndexOptions=Index vector (dialog)  Indices=[19]  OutputSizes=1
  Selector3 : Selector  InputPortWidth=21  IndexOptions=Index vector (dialog)  Indices=[12]  OutputSizes=1
  Selector4 : Selector  InputPortWidth=21  IndexOptions=Index vector (dialog)  Indices=[17]  OutputSizes=1
  Selector5 : Selector  InputPortWidth=21  IndexOptions=Index vector (dialog)  Indices=[18]  OutputSizes=1
  Selector6 : Selector  InputPortWidth=21  IndexOptions=Index vector (dialog)  Indices=[16]  OutputSizes=1
  Selector7 : Selector  InputPortWidth=21  IndexOptions=Index vector (dialog)  Indices=[11]  OutputSizes=1
  Selector8 : Selector  InputPortWidth=21  IndexOptions=Index vector (dialog)  Indices=[10]  OutputSizes=1
  Selector9 : Selector  InputPortWidth=21  IndexOptions=Index vector (dialog)  Indices=[4]  OutputSizes=1
  Traj2State2 : Subsystem
  state : Outport
  wires:
  Mux9/1 -> ApplyRotation2/2, ApplyRotation1/2, ApplyRotation/2
  Selector20/1 -> Mux9/3
  Selector19/1 -> Mux9/2
  Selector21/1 -> Mux9/1
  Selector5/1 -> Mux8/3
  Selector4/1 -> Mux8/2
  Selector6/1 -> Mux8/1
  Mux8/1 -> Mux/6
  Mux6/1 -> ApplyRotation2/1
  Mux7/1 -> Mux/4
  ApplyRotation2/1 -> Mux/5
  Selector3/1 -> Mux7/3
  Selector7/1 -> Mux7/2
  Selector8/1 -> Mux7/1
  Mux4/1 -> Mux/2
  Mux5/1 -> ApplyRotation1/1
  ApplyRotation1/1 -> Mux/3
  Mux2/1 -> ApplyRotation/1
  ApplyRotation/1 -> Mux/1
  Selector2/1 -> Mux4/3
  Selector1/1 -> Mux4/2
  Selector9/1 -> Mux4/1
  Selector17/1 -> Mux6/3
  Selector16/1 -> Mux6/2
  Selector18/1 -> Mux6/1
  Selector14/1 -> Mux5/3
  Selector13/1 -> Mux5/2
  Selector15/1 -> Mux5/1
  Selector11/1 -> Mux2/3
  Selector10/1 -> Mux2/2
  Selector12/1 -> Mux2/1
  Mux3/1 -> Selector8/1, Selector7/1, Selector3/1, Selector18/1, Selector16/1, Selector17/1, Selector6/1, Selector20/1, Selector19/1, Selector21/1, Selector5/1, Selector4/1, Selector14/1, Selector13/1, Selector15/1, Selector2/1, Selector1/1, Selector9/1, Selector12/1, Selector10/1, Selector11/1
  Mux1/1 -> Add2/2
  Constant/1 -> Mux1/2
  Add2/1 -> Traj2State2/1(traj)
  Selector/1 -> Mux3/2
  Traj Org/1 -> Mux3/1
  Offset/1 -> Mux1/1, Selector/1
  Mux/1 -> Add2/1
  Traj2State2/1(state) -> state/1

== system Demo_TrafficFlow_cs/Motorcyclist_1/AIR_5_Demux/Activate_Switch ==
  BeamID : Inport
  Heading : Inport  Port=2
  Constant : Constant  Value=0
  Product1 : Product  RndMeth=Zero
  Relational Operator : RelationalOperator  Operator=~=  OutDataTypeStr=boolean
  On/Off : Outport
  wires:
  Constant/1 -> Relational Operator/1
  Relational Operator/1 -> Product1/1
  BeamID/1 -> Relational Operator/2
  Product1/1 -> On/Off/1
  Heading/1 -> Product1/2

== system Demo_TrafficFlow_cs/Motorcyclist_1/AIR_5_Demux/Signal2ArrayOfSignals1 ==
  note: Set mask parameter VECTORLENGTH
      |  to MAXMESSAGES
  note: Initial state of Previous vector block
      | set to zeros(VECTORLENGTH,1)
  LoopIndex : Inport
  Element : Inport  Port=2
  Assignment : Assignment  IndexOptions=Index vector (port)  Indices=1  OutputSizes=1
  Previous vector : Memory  X0=zeros(VECTORLENGTH,1)  InheritSampleTime=on
  ArrayofElements : Outport
  wires:
  Element/1 -> Assignment/2
  LoopIndex/1 -> Assignment/3
  Previous vector/1 -> Assignment/1
  Assignment/1 -> ArrayofElements/1, Previous vector/1

== system Demo_TrafficFlow_cs/Motorcyclist_1/AIR_5_Demux/Signal2ArrayOfSignals2 ==
  note: Set mask parameter VECTORLENGTH
      |  to MAXMESSAGES
  note: Initial state of Previous vector block
      | set to zeros(VECTORLENGTH,1)
  LoopIndex : Inport
  Element : Inport  Port=2
  Assignment : Assignment  IndexOptions=Index vector (port)  Indices=1  OutputSizes=1
  Previous vector : Memory  X0=zeros(VECTORLENGTH,1)  InheritSampleTime=on
  ArrayofElements : Outport
  wires:
  Element/1 -> Assignment/2
  LoopIndex/1 -> Assignment/3
  Previous vector/1 -> Assignment/1
  Assignment/1 -> ArrayofElements/1, Previous vector/1

== system Demo_TrafficFlow_cs/Motorcyclist_1/AIR_5_Demux/Signal2ArrayOfSignals3 ==
  note: Set mask parameter VECTORLENGTH
      |  to MAXMESSAGES
  note: Initial state of Previous vector block
      | set to zeros(VECTORLENGTH,1)
  LoopIndex : Inport
  Element : Inport  Port=2
  Assignment : Assignment  IndexOptions=Index vector (port)  Indices=1  OutputSizes=1
  Previous vector : Memory  X0=zeros(VECTORLENGTH,1)  InheritSampleTime=on
  ArrayofElements : Outport
  wires:
  Element/1 -> Assignment/2
  LoopIndex/1 -> Assignment/3
  Previous vector/1 -> Assignment/1
  Assignment/1 -> ArrayofElements/1, Previous vector/1

== system Demo_TrafficFlow_cs/Motorcyclist_1/AIR_5_Demux/Signal2ArrayOfSignals4 ==
  note: Set mask parameter VECTORLENGTH
      |  to MAXMESSAGES
  note: Initial state of Previous vector block
      | set to zeros(VECTORLENGTH,1)
  LoopIndex : Inport
  Element : Inport  Port=2
  Assignment : Assignment  IndexOptions=Index vector (port)  Indices=1  OutputSizes=1
  Previous vector : Memory  X0=zeros(VECTORLENGTH,1)  InheritSampleTime=on
  ArrayofElements : Outport
  wires:
  Element/1 -> Assignment/2
  LoopIndex/1 -> Assignment/3
  Previous vector/1 -> Assignment/1
  Assignment/1 -> ArrayofElements/1, Previous vector/1

== system Demo_TrafficFlow_cs/Motorcyclist_1/AIR_5_Demux/Signal2ArrayOfSignals5 ==
  note: Set mask parameter VECTORLENGTH
      |  to MAXMESSAGES
  note: Initial state of Previous vector block
      | set to zeros(VECTORLENGTH,1)
  LoopIndex : Inport
  Element : Inport  Port=2
  Assignment : Assignment  IndexOptions=Index vector (port)  Indices=1  OutputSizes=1
  Previous vector : Memory  X0=zeros(VECTORLENGTH,1)  InheritSampleTime=on
  ArrayofElements : Outport
  wires:
  Element/1 -> Assignment/2
  LoopIndex/1 -> Assignment/3
  Previous vector/1 -> Assignment/1
  Assignment/1 -> ArrayofElements/1, Previous vector/1
